simulink model "non_linear_MBS_PF_final"  (R2021b)
settings: Solver=ode15s  StartTime=0  StopTime=SimTime  FixedStep=.00001  RelTol=1e-3
values from neighbouring files (symbols this model uses):
  A_ratio = 1.41    [non_linear_param_MBS_PF_final.m]    (also in non_linear_param_final.m)
  Annulus_area = Bore_area-Rod_area    (= 2356.19449019)    [non_linear_param_MBS_PF_final.m]    (also in non_linear_param_final.m)
  beta_F = 12000e5    (= 1200000000)    [non_linear_param_MBS_PF_final.m]    (also in non_linear_param_final.m)
  Bore_area = pi/4*Bore_diameter^2    (= 3318.30724035)    [non_linear_param_MBS_PF_final.m]    (also in non_linear_param_final.m)
  cyl_c_end = 9e8    (= 900000000)    [non_linear_param_MBS_PF_final.m]    (also in non_linear_param_final.m)
  cyl_c_visc = 1500    [non_linear_param_MBS_PF_final.m]    (also in non_linear_param_final.m)
  cyl_CH_stat = 1/50    (= 0.02)    [non_linear_param_MBS_PF_final.m]    (also in non_linear_param_final.m)
  cyl_F_coul = 75    [non_linear_param_MBS_PF_final.m]    (also in non_linear_param_final.m)
  cyl_FH_stat = 500    [non_linear_param_MBS_PF_final.m]    (also in non_linear_param_final.m)
  cyl_gamma = 250    [non_linear_param_MBS_PF_final.m]    (also in non_linear_param_final.m)
  cyl_k_end = 6e9    (= 6000000000)    [non_linear_param_MBS_PF_final.m]    (also in non_linear_param_final.m)
  cyl_KLi = 0    [non_linear_param_MBS_PF_final.m]    (also in non_linear_param_final.m)
  d_eff_long = sqrt(2*J_sec_long*k_eff_long)    [non_linear_param_MBS_PF_final.m]    (also in non_linear_param_final.m)
  d_eff_short = sqrt(2*J_sec_short*k_eff_short)    [non_linear_param_MBS_PF_final.m]    (also in non_linear_param_final.m)
  DB = 0    [non_linear_param_MBS_PF_final.m]    (also in non_linear_param_final.m = -0.114)
  DR = 1    [non_linear_param_MBS_PF_final.m]    (also in non_linear_param_final.m)
  dr_dcv = 0.7    [non_linear_param_MBS_PF_final.m]    (also in non_linear_param_final.m)
  Eff_Bulk_On = 1    [non_linear_param_MBS_PF_final.m]    (also in non_linear_param_final.m)
  eta_air = 0.7e-2    (= 0.007)    [non_linear_param_MBS_PF_final.m]    (also in non_linear_param_final.m)
  k_cv = 15    [non_linear_param_MBS_PF_final.m]    (also in non_linear_param_final.m)
  k_DCV_PA = 7.85    [non_linear_param_MBS_PF_final.m]    (also in non_linear_param_final.m)
  k_DCV_PB = 7.55    [non_linear_param_MBS_PF_final.m]    (also in non_linear_param_final.m)
  k_DCV_T = 7.85    [non_linear_param_MBS_PF_final.m]    (also in non_linear_param_final.m)
  k_eff_long = E*I/I_sec_long    (= 3050381.94041)    [non_linear_param_MBS_PF_final.m]    (also in non_linear_param_final.m)
  k_eff_short = E*I/I_sec_short    (= 13451432.5427)    [non_linear_param_MBS_PF_final.m]    (also in non_linear_param_final.m)
  k_ocv = 15    [non_linear_param_MBS_PF_final.m]    (also in non_linear_param_final.m)
  K_oil = 1.5e4*0+12000    (= 12000)    [non_linear_param_MBS_PF_final.m]    (also in non_linear_param_final.m)
  k_tanh = 0.005    [non_linear_param_MBS_PF_final.m]    (also in non_linear_param_final.m)
  kappa_air = 1.4    [non_linear_param_MBS_PF_final.m]    (also in non_linear_param_final.m)
  kHP = C*kF_pf_Up    (= 2.90878e-12)    [non_linear_param_MBS_PF_final.m]
  kI = (0.54*ku)/Ti    (= 0.048)    [non_linear_param_MBS_PF_final.m]
  kP = 0.45*ku    (= 0.018)    [non_linear_param_MBS_PF_final.m]
  kq_xS_PB = 4.8946e-04    (= 0.00048946)    [non_linear_param_MBS_PF_final.m]
  KR = 1    [non_linear_param_MBS_PF_final.m]    (also in non_linear_param_final.m)
  mu_ocv = 10    [non_linear_param_MBS_PF_final.m]    (also in non_linear_param_final.m = 3)
  muC = (Bore_diameter^2)/(Bore_diameter^2-Rod_diameter^2)    (= 1.40833333333)    [non_linear_param_MBS_PF_final.m]
  OL_pa = -0.114    [non_linear_param_MBS_PF_final.m]    (also in non_linear_param_final.m)
  OL_pb = 0.114    [non_linear_param_MBS_PF_final.m]    (also in non_linear_param_final.m)
  p0_A = 62.79    [non_linear_param_MBS_PF_final.m]    (also in non_linear_param_final.m)
  p0_A1 = 1.08    [non_linear_param_MBS_PF_final.m]    (also in non_linear_param_final.m)
  p0_B = 1.213    [non_linear_param_MBS_PF_final.m]    (also in non_linear_param_final.m)
  p_cv_fo = 1    [non_linear_param_MBS_PF_final.m]    (also in non_linear_param_final.m)
  p_cv_set = 2.8    [non_linear_param_MBS_PF_final.m]    (also in non_linear_param_final.m)
  p_ocv_fo = 350    [non_linear_param_MBS_PF_final.m]    (also in non_linear_param_final.m)
  p_ocv_set = 196    [non_linear_param_MBS_PF_final.m]    (also in non_linear_param_final.m = 150)
  p_pc_set = 7    [non_linear_param_MBS_PF_final.m]    (also in non_linear_param_final.m)
  p_R = 1.1    [non_linear_param_MBS_PF_final.m]    (also in non_linear_param_final.m)
  p_S = 180    [non_linear_param_MBS_PF_final.m]    (also in non_linear_param_final.m)
  patm = 1.013e5    (= 101300)    [non_linear_param_MBS_PF_final.m]    (also in non_linear_param_final.m)
  Stroke = 500    [non_linear_param_MBS_PF_final.m]    (also in non_linear_param_final.m)
  t0 = 3    [non_linear_param_MBS_PF_final.m]    (also in non_linear_param_final.m = 1)
  tau_ocv = 0.0889*0+0.001    (= 0.001)    [non_linear_param_MBS_PF_final.m]    (also in non_linear_param_final.m)
  tau_pc = tau_ocv    (= 0.001)    [non_linear_param_MBS_PF_final.m]    (also in non_linear_param_final.m)
  tHP = tF_pf_Up/(wn_pf)    (= 0.114613180516)    [non_linear_param_MBS_PF_final.m]
  tStepDown = 4.7*0+7.5    (= 7.5)    [non_linear_param_MBS_PF_final.m]    (also in non_linear_param_final.m = 4.6)
  tStepUp = 10.0    (= 10)    [non_linear_param_MBS_PF_final.m]    (also in non_linear_param_final.m = 6.2)
  tWait = 5.0    (= 5)    [non_linear_param_MBS_PF_final.m]    (also in non_linear_param_final.m = 8)
  UL_at = -0.05    [non_linear_param_MBS_PF_final.m]    (also in non_linear_param_final.m)
  UL_bt = 0.05    [non_linear_param_MBS_PF_final.m]    (also in non_linear_param_final.m)
  Up = 1    [non_linear_param_MBS_PF_final.m]    (also in non_linear_param_final.m)
  uS_sp = -0.4    [non_linear_param_MBS_PF_final.m]    (also in non_linear_param_final.m = -0.55)
  V_CH_A = 1.0e-03    (= 0.001)    [non_linear_param_MBS_PF_final.m]    (also in non_linear_param_final.m)
  V_CH_A1 = 0.1e-03    (= 0.0001)    [non_linear_param_MBS_PF_final.m]    (also in non_linear_param_final.m)
  V_CH_B = 1.25e-03    (= 0.00125)    [non_linear_param_MBS_PF_final.m]    (also in non_linear_param_final.m)
  wn_dcv = 30    [non_linear_param_MBS_PF_final.m]    (also in non_linear_param_final.m)
undefined symbols (not defined in the file or in its folder): sigbuilder_block, smiData, uS0, X, Y, Z
libraries: sm_lib x189, nesl_utility x4, eeGeneralControl x2
(part 3 of 6)

== system non_linear_MBS_PF_final/Comparison Exp1/Large valve opening = 0.5 Down Only ==
  Demux : Demux  Tag=STV Demux  Outputs=9
      settings: DisplayOption=none
  FromWs : FromWorkspace  Tag=STV FromWs  VariableName=tuvar
  Imported_Signal 1 : Outport  Tag=STV Outport  VectorParamsAs1DForOutWhenUnconnected=off
  Imported_Signal 2 : Outport  Tag=STV Outport  Port=2  VectorParamsAs1DForOutWhenUnconnected=off
  Imported_Signal 3 : Outport  Tag=STV Outport  Port=3  VectorParamsAs1DForOutWhenUnconnected=off
  Imported_Signal 4 : Outport  Tag=STV Outport  Port=4  VectorParamsAs1DForOutWhenUnconnected=off
  Imported_Signal 5 : Outport  Tag=STV Outport  Port=5  VectorParamsAs1DForOutWhenUnconnected=off
  Imported_Signal 6 : Outport  Tag=STV Outport  Port=6  VectorParamsAs1DForOutWhenUnconnected=off
  Imported_Signal 7 : Outport  Tag=STV Outport  Port=7  VectorParamsAs1DForOutWhenUnconnected=off
  Imported_Signal 8 : Outport  Tag=STV Outport  Port=8  VectorParamsAs1DForOutWhenUnconnected=off
  Imported_Signal 9 : Outport  Tag=STV Outport  Port=9  VectorParamsAs1DForOutWhenUnconnected=off
  wires:
  Demux/1 -> Imported_Signal 1/1
  FromWs/1 -> Demux/1
  Demux/2 -> Imported_Signal 2/1
  Demux/3 -> Imported_Signal 3/1
  Demux/4 -> Imported_Signal 4/1
  Demux/5 -> Imported_Signal 5/1
  Demux/6 -> Imported_Signal 6/1
  Demux/7 -> Imported_Signal 7/1
  Demux/8 -> Imported_Signal 8/1
  Demux/9 -> Imported_Signal 9/1

== system non_linear_MBS_PF_final/Comparison Exp1/Orginal DOwn ==
  Demux : Demux  Tag=STV Demux  Outputs=9
      settings: DisplayOption=none
  FromWs : FromWorkspace  Tag=STV FromWs  VariableName=tuvar
  Imported_Signal 1 : Outport  Tag=STV Outport  VectorParamsAs1DForOutWhenUnconnected=off
  Imported_Signal 2 : Outport  Tag=STV Outport  Port=2  VectorParamsAs1DForOutWhenUnconnected=off
  Imported_Signal 3 : Outport  Tag=STV Outport  Port=3  VectorParamsAs1DForOutWhenUnconnected=off
  Imported_Signal 4 : Outport  Tag=STV Outport  Port=4  VectorParamsAs1DForOutWhenUnconnected=off
  Imported_Signal 5 : Outport  Tag=STV Outport  Port=5  VectorParamsAs1DForOutWhenUnconnected=off
  Imported_Signal 6 : Outport  Tag=STV Outport  Port=6  VectorParamsAs1DForOutWhenUnconnected=off
  Imported_Signal 7 : Outport  Tag=STV Outport  Port=7  VectorParamsAs1DForOutWhenUnconnected=off
  Imported_Signal 8 : Outport  Tag=STV Outport  Port=8  VectorParamsAs1DForOutWhenUnconnected=off
  Imported_Signal 9 : Outport  Tag=STV Outport  Port=9  VectorParamsAs1DForOutWhenUnconnected=off
  wires:
  Demux/1 -> Imported_Signal 1/1
  FromWs/1 -> Demux/1
  Demux/2 -> Imported_Signal 2/1
  Demux/3 -> Imported_Signal 3/1
  Demux/4 -> Imported_Signal 4/1
  Demux/5 -> Imported_Signal 5/1
  Demux/6 -> Imported_Signal 6/1
  Demux/7 -> Imported_Signal 7/1
  Demux/8 -> Imported_Signal 8/1
  Demux/9 -> Imported_Signal 9/1

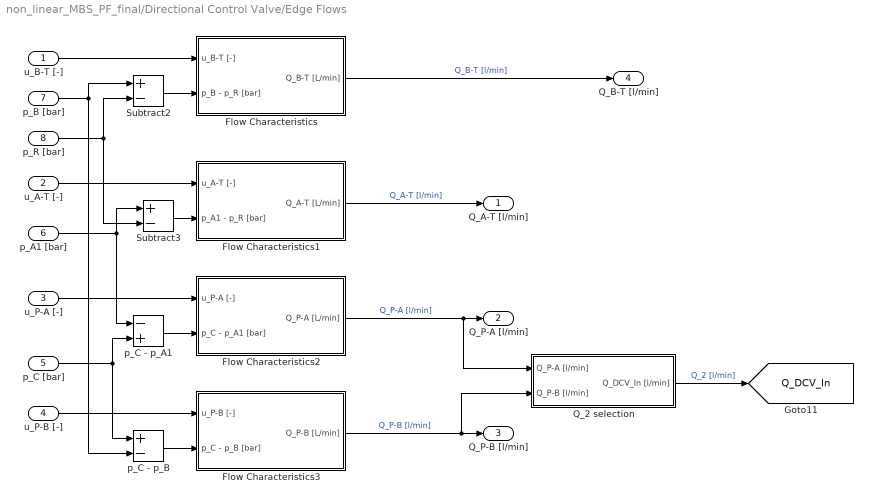
== system non_linear_MBS_PF_final/Directional Control Valve/Edge Flows ==
  u_B-T [-] : Inport
  u_A-T [-] : Inport  Port=2
  u_P-A [-] : Inport  Port=3
  u_P-B [-] : Inport  Port=4
  p_C [bar] : Inport  Port=5
  p_A1 [bar] : Inport  Port=6
  p_B [bar] : Inport  Port=7
  p_R [bar] : Inport  Port=8
  Flow Characteristics : Subsystem
  Flow Characteristics1 : Subsystem
  Flow Characteristics2 : Subsystem
  Flow Characteristics3 : Subsystem
  Q_2 selection : Subsystem
  Subtract2 : Sum  Inputs=+-
  Subtract3 : Sum  Inputs=+-
  p_C - p_A1 : Sum  Inputs=-+
  p_C - p_B : Sum  Inputs=+-
  Q_A-T [l/min] : Outport  VectorParamsAs1DForOutWhenUnconnected=off
  Q_P-A [l/min] : Outport  Port=2  VectorParamsAs1DForOutWhenUnconnected=off
  Q_P-B [l/min] : Outport  Port=3  VectorParamsAs1DForOutWhenUnconnected=off
  Q_B-T [l/min] : Outport  Port=4  VectorParamsAs1DForOutWhenUnconnected=off
  tag scope: Q_DCV_In=global
  wires:
  p_C [bar]/1 -> p_C - p_A1/2, p_C - p_B/1
  u_A-T [-]/1 -> Flow Characteristics1/1(u_A-T [-])
  p_A1 [bar]/1 -> Subtract3/1, p_C - p_A1/1
  p_B [bar]/1 -> Subtract2/1, p_C - p_B/2
  p_C - p_B/1 -> Flow Characteristics3/2(p_C - p_B [bar])
  u_P-A [-]/1 -> Flow Characteristics2/1(u_P-A [-])
  p_C - p_A1/1 -> Flow Characteristics2/2(p_C - p_A1 [bar])
  u_P-B [-]/1 -> Flow Characteristics3/1(u_P-B [-])
  p_R [bar]/1 -> Subtract3/2, Subtract2/2
  Flow Characteristics1/1(Q_A-T [L/min]) -> Q_A-T [l/min]/1  "Q_A-T [l/min]"
  Flow Characteristics/1(Q_B-T [L/min]) -> Q_B-T [l/min]/1  "Q_B-T [l/min]"
  Q_2 selection/1(Q_DCV_In [l/min]) -> [Q_DCV_In]  "Q_2 [l/min]"
  Flow Characteristics3/1(Q_P-B [L/min]) -> Q_2 selection/2(Q_P-B [l/min]), Q_P-B [l/min]/1  "Q_P-B [l/min]"
  Flow Characteristics2/1(Q_P-A [L/min]) -> Q_2 selection/1(Q_P-A [l/min]), Q_P-A [l/min]/1  "Q_P-A [l/min]"
  Subtract2/1 -> Flow Characteristics/2(p_B - p_R [bar])
  u_B-T [-]/1 -> Flow Characteristics/1(u_B-T [-])
  Subtract3/1 -> Flow Characteristics1/2(p_A1 - p_R [bar])

== system non_linear_MBS_PF_final/Directional Control Valve/Edge Openings ==
  u_dcv [-] : Inport
  Edge A-T : Subsystem
  Edge B-T : Subsystem
  Edge P-A : Subsystem
  Edge P-B : Subsystem
  u_B-T [-] : Outport  VectorParamsAs1DForOutWhenUnconnected=off
  u_A-T [-] : Outport  Port=2  VectorParamsAs1DForOutWhenUnconnected=off
  u_P-A [-] : Outport  Port=3  VectorParamsAs1DForOutWhenUnconnected=off
  u_P-B [-] : Outport  Port=4  VectorParamsAs1DForOutWhenUnconnected=off
  wires:
  Edge A-T/1(u_A-T [-]) -> u_A-T [-]/1  "u_A-T [-]"
  Edge B-T/1(u_B-T [-]) -> u_B-T [-]/1  "u_B-T [-]"
  u_dcv [-]/1 -> Edge P-A/1(u_dcv [-]), Edge P-B/1(u_dcv [-]), Edge A-T/1(u_dcv [-]), Edge B-T/1(u_dcv [-])  "[-]"
  Edge P-A/1(u_P-A [-]) -> u_P-A [-]/1  "u_P-A [-]"
  Edge P-B/1(u_P-B [-]) -> u_P-B [-]/1  "u_P-B [-]"

== system non_linear_MBS_PF_final/Directional Control Valve/Load-Sensing Pressure ==
  pB : Inport
  pA1 : Inport  Port=2
  uDCV : Inport  Port=3
  Constant : Constant  Value=0
  Switch : Switch  Criteria=u2 ~= 0
  Switch1 : Switch  Criteria=u2 > Threshold
  pLS : Outport  VectorParamsAs1DForOutWhenUnconnected=off
  wires:
  pA1/1 -> Switch1/3
  Constant/1 -> Switch/3
  Switch1/1 -> Switch/1
  Switch/1 -> pLS/1
  uDCV/1 -> Switch1/2, Switch/2
  pB/1 -> Switch1/1

== system non_linear_MBS_PF_final/Directional Control Valve/Valve Dynamics ==
  note: 2nd order TF for
      | DCV dynamics
  u_dcv_ref [-] : Inport
  2nd order TF : TransferFcn  Denominator="[1/(wn_dcv^2) 2*dr_dcv/wn_dcv  1]"
  u_dcv [-] : Outport  VectorParamsAs1DForOutWhenUnconnected=off
  tag scope: u_dcv=global
  wires:
  u_dcv_ref [-]/1 -> 2nd order TF/1  "u_dcv_ref [-]"
  2nd order TF/1 -> [u_dcv], u_dcv [-]/1  "u_dcv [-]"

== system non_linear_MBS_PF_final/Monitoring/Subsystem ==
  Constant1 : Constant  Value=A_ratio
  Divide3 : Product  Inputs=**
  Gain15 : Gain  Gain=-1
  Q_A exp [L/min] : Outport  VectorParamsAs1DForOutWhenUnconnected=off
  wires:
  [Q_B_exp] -> Divide3/1
  Gain15/1 -> Q_A exp [L/min]/1  "Q_A exp [L/min]"
  Constant1/1 -> Divide3/2
  Divide3/1 -> Gain15/1

== system non_linear_MBS_PF_final/Over-Centre Valve/Capasitance ==
  Bulk Modulus : Subsystem
  Constant : Constant  Value=V_CH_A1
  Divide : Product  Inputs=*/
  "CH_A1 " : Outport  VectorParamsAs1DForOutWhenUnconnected=off
  wires:
  Bulk Modulus/1(K_oil) -> Divide/2  "[bar]"
  Constant/1 -> Divide/1  "[m^3]"
  Divide/1 -> "CH_A1 "/1

== system non_linear_MBS_PF_final/Over-Centre Valve/Check Valve ==
  p_A [bar] : Inport
  p_A1 [bar] : Inport  Port=2
  Abs : Abs  ZeroCross=off
  Constant : Constant  Value=k_cv
  Product : Product  Inputs=****
  Saturation : Saturate  UpperLimit=1  LowerLimit=0  ZeroCross=off
  Sign : Subsystem
  Sqrt : Sqrt  SaturateOnIntegerOverflow=on
  Sum : Sum  Inputs=+-
  Sum1 : Sum  Inputs=+-
  division by p_cv_fo : Gain  Gain=1/p_cv_fo
  p_cv_set [bar] : Constant  Value=p_cv_set
  Q_cv [l/min] : Outport  VectorParamsAs1DForOutWhenUnconnected=off
  wires:
  p_cv_set [bar]/1 -> Sum1/2
  Sum/1 -> Abs/1, Sum1/1, Sign/1(In)  "p2-p3 [bar]"
  p_A [bar]/1 -> Sum/2
  p_A1 [bar]/1 -> Sum/1
  division by p_cv_fo/1 -> Saturation/1  "u_cv [-]"
  Sum1/1 -> division by p_cv_fo/1
  Constant/1 -> Product/1  "[l/min/bar^0.5]"
  Sqrt/1 -> Product/2  "[bar^0.5]"
  Product/1 -> Q_cv [l/min]/1
  Abs/1 -> Sqrt/1
  Sign/1(Out) -> Product/4
  Saturation/1 -> Product/3

== system non_linear_MBS_PF_final/Over-Centre Valve/Over-Centre Valve ==
  u_ocv [-] : Inport
  p_A [bar] : Inport  Port=2
  p_A1 [bar] : Inport  Port=3
  Abs : Abs  ZeroCross=off
  Product : Product  Inputs=****
  Sign : Subsystem
  Sqrt : Sqrt  SaturateOnIntegerOverflow=on
  k_ocv [l/min/bar^0.5] : Constant  Value=k_ocv
  p3-p2 : Sum  Inputs=+-
  "[l/min]" : Outport  VectorParamsAs1DForOutWhenUnconnected=off
  wires:
  k_ocv [l/min/bar^0.5]/1 -> Product/3
  p3-p2/1 -> Sign/1(In), Abs/1  "p3-p2 [bar]"
  Sqrt/1 -> Product/2  "[bar^0.5]"
  Product/1 -> "[l/min]"/1  "[l/min]"
  Sign/1(Out) -> Product/4
  Abs/1 -> Sqrt/1
  u_ocv [-]/1 -> Product/1
  p_A1 [bar]/1 -> p3-p2/2
  p_A [bar]/1 -> p3-p2/1

== system non_linear_MBS_PF_final/Over-Centre Valve/Pressure Equillibrium ==
  p_B [bar] : Inport
  p_A [bar] : Inport  Port=2
  Division by p_ocv_fo [bar] : Gain  Gain=1/p_ocv_fo
  Pilot ratio [-] : Gain  Gain=mu_ocv
  Saturation : Saturate  UpperLimit=1  LowerLimit=0  ZeroCross=off
  Scope : Scope
  Sum : Sum  Inputs=++-
  Valve Dynamics : TransferFcn  Denominator=[tau_ocv 1]
  p_ocv_set [bar] : Constant  Value=p_ocv_set
  u_ocv [-] : Outport  VectorParamsAs1DForOutWhenUnconnected=off
  wires:
  Sum/1 -> Division by p_ocv_fo [bar]/1  "[bar]"
  Pilot ratio [-]/1 -> Sum/1
  p_ocv_set [bar]/1 -> Sum/3
  p_B [bar]/1 -> Pilot ratio [-]/1
  p_A [bar]/1 -> Sum/2
  Division by p_ocv_fo [bar]/1 -> Saturation/1  "u_ocv_ref [-]"
  Saturation/1 -> Valve Dynamics/1
  Valve Dynamics/1 -> Scope/1, u_ocv [-]/1  "[-]"

== system non_linear_MBS_PF_final/Subsystem/CH Bore Side ==
  x [m] : Inport
  Bulk Modulus : Subsystem
  Constant4 : Constant  Value=V_CH_A
  Divide2 : Product  Inputs=*/
  Gain1 : Gain  Gain=Bore_area
  Gain2 : Gain  Gain=1/(10)^9
  Gain3 : Gain  Gain=1000
  Sum3 : Sum
  CH_A : Outport  VectorParamsAs1DForOutWhenUnconnected=off
  wires:
  Sum3/1 -> Divide2/1  "[m^3]"
  x [m]/1 -> Gain3/1
  Constant4/1 -> Sum3/1  "[m^3]"
  Gain1/1 -> Gain2/1  "[mm^3]"
  Gain3/1 -> Gain1/1  "[mm]"
  Gain2/1 -> Sum3/2  "[m^3]"
  Divide2/1 -> CH_A/1
  Bulk Modulus/1(K_oil) -> Divide2/2  "[bar]"

== system non_linear_MBS_PF_final/Subsystem/CH Rod Side ==
  x [m] : Inport
  Bulk Modulus : Subsystem
  Constant1 : Constant  Value=Stroke
  Constant4 : Constant  Value=V_CH_B
  Divide2 : Product  Inputs=*/
  Gain1 : Gain  Gain=Annulus_area
  Gain2 : Gain  Gain=1/(10)^9
  Gain4 : Gain  Gain=1000
  Sum2 : Sum  Inputs=+-
  Sum3 : Sum
  CH_B : Outport  VectorParamsAs1DForOutWhenUnconnected=off
  wires:
  Sum3/1 -> Divide2/1  "[m^3]"
  Constant1/1 -> Sum2/1  "[mm]"
  x [m]/1 -> Gain4/1
  Sum2/1 -> Gain1/1
  Constant4/1 -> Sum3/1  "[m^3]"
  Gain1/1 -> Gain2/1  "[mm^3]"
  Gain4/1 -> Sum2/2  "[mm]"
  Gain2/1 -> Sum3/2  "[m^3]"
  Divide2/1 -> CH_B/1
  Bulk Modulus/1(K_oil) -> Divide2/2

== system non_linear_MBS_PF_final/Subsystem/Cylinder forces ==
  v_cyl [m/s] : Inport
  F_hyd [N] : Inport  Port=2
  x _cyl [m] : Inport  Port=3
  Add : Sum  Inputs=+-+-
  Constant : Constant  Value=0
  Lower Stroke Limit : Subsystem
  Manual Switch : ManualSwitch
  Stribeck Friction1 : Subsystem
  Upper Stroke Limit : Subsystem
  F_piston [N] : Outport  VectorParamsAs1DForOutWhenUnconnected=off
  wires:
  Lower Stroke Limit/1(F_cyl_contact [N]) -> Add/3
  Upper Stroke Limit/1(F_cyl_contact [N]) -> Add/2
  x _cyl [m]/1 -> Upper Stroke Limit/1(x_cyl [m]), Lower Stroke Limit/1(x_cyl [m])
  Add/1 -> F_piston [N]/1  "Bm_Cyl_F"
  Stribeck Friction1/1(F [N]) -> Manual Switch/1
  v_cyl [m/s]/1 -> Stribeck Friction1/1(v_cyl [m/s]), Lower Stroke Limit/2(v_cyl [m]), Upper Stroke Limit/2(v_cyl [m/s])
  Manual Switch/1 -> Add/4
  F_hyd [N]/1 -> Add/1
  Constant/1 -> Manual Switch/2

== system non_linear_MBS_PF_final/Subsystem/Internal Leakages ==
  Dp [bar] : Inport  PortDimensions=1
  Conv.  1 : Gain  Gain=cyl_KLi
  Product : Product  RndMeth=Zero
  Sign : Signum
  Q_L [L/min] : Outport  PortDimensions=1  VectorParamsAs1DForOutWhenUnconnected=off
  wires:
  Dp [bar]/1 -> Conv.  1/1, Sign/1
  Product/1 -> Q_L [L/min]/1
  Conv.  1/1 -> Product/1
  Sign/1 -> Product/2  "[-]"

== system non_linear_MBS_PF_final/Subsystem1/VCC_Step_Function_Validation ==
  Enable : Inport  OutDataTypeStr=boolean
  u_SP : Inport  Port=2
  uS0 : Inport  Port=3
  DB : Inport  Port=4
  CyclicTime : Inport  Port=5
  t0 : Inport  Port=6
  tHold1 : Inport  Port=7
  tStep : Inport  Port=8
  tHold2 : Inport  Port=9
  Constant : Constant  Value=0
  StepFunction : MATLAB Function  TreatAsAtomicUnit=on
  Switch : Switch  Criteria=u2 > Threshold
  u_ref : Outport  VectorParamsAs1DForOutWhenUnconnected=off
  EndTime : Outport  Port=2  VectorParamsAs1DForOutWhenUnconnected=off
  wires:
  u_SP/1 -> StepFunction/1(u_SP)
  tStep/1 -> StepFunction/7(t2)
  tHold1/1 -> StepFunction/6(t1)
  t0/1 -> StepFunction/5(t0)
  CyclicTime/1 -> StepFunction/4(t)
  StepFunction/2(T) -> EndTime/1
  uS0/1 -> StepFunction/2(uS0)
  tHold2/1 -> StepFunction/8(t3)
  DB/1 -> StepFunction/3(DB)
  StepFunction/1(y) -> Switch/1
  Switch/1 -> u_ref/1
  Constant/1 -> Switch/3
  Enable/1 -> Switch/2

== system non_linear_MBS_PF_final/Subsystem2/Efficiency v1 ==
  Pin : Inport
  Pout : Inport  Port=2
  1 : Scope
  2 : Scope
  3 : Scope
  Fcn4 : Fcn  Expr=u(2)/(u(1)+eps)
  Integrator2 : Integrator  LowerSaturationLimit=0
  Mux9 : Mux  Inputs=2
  Saturation3 : Saturate  UpperLimit=inf  LowerLimit=0
  Saturation4 : Saturate  UpperLimit=1  LowerLimit=0
  eta_io : Outport  VectorParamsAs1DForOutWhenUnconnected=off
  wires:
  Pin/1 -> Mux9/1
  Pout/1 -> Mux9/2
  Mux9/1 -> Saturation3/1, 1/1
  Saturation4/1 -> eta_io/1
  Fcn4/1 -> Saturation4/1
  Integrator2/1 -> Fcn4/1, 3/1
  Saturation3/1 -> Integrator2/1, 2/1

== system non_linear_MBS_PF_final/Test/Arm_ballast_big_4_RIGID ==
  ReferenceFrame : sm_lib/Frames and Transforms/Reference Frame
  Rigid Transform2 : sm_lib/Frames and Transforms/Rigid Transform  TranslationMethod=Cartesian  TranslationStandardOffset=0  TranslationLengthUnit=mm  TranslationStandardAxis=+Z  TranslationCartesianOffset=[0 6.5 -(609.43814785-105.00)]  TranslationROffset=0  TranslationROffsetUnits=m  TranslationZOffset=0  TranslationZOffsetUnits=m  TranslationThetaOffset=0  TranslationThetaOffsetUnits=deg  RotationMethod=ArbitraryAxis  RotationAngleUnits=deg  RotationStandardAxis=+Z  RotationAngle=0  RotationArbitraryAxis=[0 1 0]  FollAlignAxisA=+X  BaseAlignAxisA=+Y  FollAlignAxisB=+Y  BaseAlignAxisB=+Z  RotationSequenceAxes=FollowerAxes  RotationSequence=XYX  RotationSequenceAngles=[0 0 0]  RotationSequenceAnglesUnits=deg  RotationMatrix=[1 0 0; 0 1 0; 0 0 1]
  Rigid Transform3 : sm_lib/Frames and Transforms/Rigid Transform  TranslationMethod=Cartesian  TranslationStandardOffset=0  TranslationLengthUnit=mm  TranslationStandardAxis=+Z  TranslationCartesianOffset=[0 0 105]  TranslationROffset=0  TranslationROffsetUnits=m  TranslationZOffset=0  TranslationZOffsetUnits=m  TranslationThetaOffset=0  TranslationThetaOffsetUnits=deg  RotationMethod=ArbitraryAxis  RotationAngleUnits=deg  RotationStandardAxis=+Z  RotationAngle=0  RotationArbitraryAxis=[0 1 0]  FollAlignAxisA=+X  BaseAlignAxisA=+Y  FollAlignAxisB=+Y  BaseAlignAxisB=+Z  RotationSequenceAxes=FollowerAxes  RotationSequence=XYX  RotationSequenceAngles=[0 0 0]  RotationSequenceAnglesUnits=deg  RotationMatrix=[1 0 0; 0 1 0; 0 0 1]
  Solid : sm_lib/Body Elements/File Solid  ExtGeomFileName=Arm_ballast_big_Default_sldprt.STEP  UnitType=FromFile  ExtGeomFileUnits=m  StepReaderType=OCC  ExportConvexHullGeometry=off  InertiaType=CalculateFromGeometry  Mass=327.5000  MassUnits=kg  CenterOfMass=smiData.Solid(23).CoM  CenterOfMassUnits=mm  MomentsOfInertia=smiData.Solid(23).MoI  MomentsOfInertiaUnits=kg*mm^2  ProductsOfInertia=smiData.Solid(23).PoI  ProductsOfInertiaUnits=kg*mm^2  BasedOnType=Mass  Density=10000  DensityUnits=kg/m^3  DoExposeReferenceFrame=on  SerializedFrames=<Frames/>  GraphicType=FromGeometry  MarkerShape=Sphere  MarkerSize=10  GraphicVisPropType=SimpleVisualProperties  GraphicDiffuseColor=[1.0 0.8 0.0]  GraphicSpecularColor=[0.5 0.5 0.5 1.0]  GraphicAmbientColor=[0.15 0.15 0.15 1.0]  GraphicEmissiveColor=[0.0 0.0 0.0 1.0]  GraphicShininess=75  GraphicOpacity=smiData.Solid(23).opacity
  F : PMIOPort  Side=Left
  F1 : PMIOPort  Port=2  Side=Right
  connections:
  ReferenceFrame/RConn1 -- Rigid Transform2/LConn1 -- Rigid Transform3/LConn1 -- Solid/RConn1
  F/RConn1 -- Rigid Transform2/RConn1
  Rigid Transform3/RConn1 -- F1/RConn1

== system non_linear_MBS_PF_final/Test/Arm_long_1_RIGID ==
  ReferenceFrame2 : sm_lib/Frames and Transforms/Reference Frame
  Rigid Transform : sm_lib/Frames and Transforms/Rigid Transform  TranslationMethod=Cartesian  TranslationStandardOffset=0  TranslationLengthUnit=m  TranslationStandardAxis=+Z  TranslationCartesianOffset=[0 0 0.6094]  TranslationROffset=0  TranslationROffsetUnits=m  TranslationZOffset=0  TranslationZOffsetUnits=m  TranslationThetaOffset=0  TranslationThetaOffsetUnits=deg  RotationMethod=ArbitraryAxis  RotationAngleUnits=deg  RotationStandardAxis=+Z  RotationAngle=90  RotationArbitraryAxis=[0 1 0]  FollAlignAxisA=+X  BaseAlignAxisA=+Y  FollAlignAxisB=+Y  BaseAlignAxisB=+Z  RotationSequenceAxes=FollowerAxes  RotationSequence=XYX  RotationSequenceAngles=[0 0 0]  RotationSequenceAnglesUnits=deg  RotationMatrix=[1 0 0; 0 1 0; 0 0 1]
  Rigid Transform3 : sm_lib/Frames and Transforms/Rigid Transform  TranslationMethod=Cartesian  TranslationStandardOffset=0  TranslationLengthUnit=m  TranslationStandardAxis=+Z  TranslationCartesianOffset=[0 0 0]  TranslationROffset=0  TranslationROffsetUnits=m  TranslationZOffset=0  TranslationZOffsetUnits=m  TranslationThetaOffset=0  TranslationThetaOffsetUnits=deg  RotationMethod=ArbitraryAxis  RotationAngleUnits=deg  RotationStandardAxis=+Z  RotationAngle=-90  RotationArbitraryAxis=[0 1 0]  FollAlignAxisA=+X  BaseAlignAxisA=+Y  FollAlignAxisB=+Y  BaseAlignAxisB=+Z  RotationSequenceAxes=FollowerAxes  RotationSequence=XYX  RotationSequenceAngles=[0 0 0]  RotationSequenceAnglesUnits=deg  RotationMatrix=[1 0 0; 0 1 0; 0 0 1]
  Solid : sm_lib/Body Elements/File Solid  ExtGeomFileName=Arm_long_Default_sldprt.STEP  UnitType=FromFile  ExtGeomFileUnits=m  StepReaderType=OCC  ExportConvexHullGeometry=off  InertiaType=CalculateFromGeometry  Mass=13.5799  MassUnits=kg  CenterOfMass=smiData.Solid(19).CoM  CenterOfMassUnits=mm  MomentsOfInertia=smiData.Solid(19).MoI  MomentsOfInertiaUnits=kg*mm^2  ProductsOfInertia=smiData.Solid(19).PoI  ProductsOfInertiaUnits=kg*mm^2  BasedOnType=Mass  Density=7800  DensityUnits=kg/m^3  DoExposeReferenceFrame=on  SerializedFrames=<Frames/>  GraphicType=FromGeometry  MarkerShape=Sphere  MarkerSize=10  GraphicVisPropType=SimpleVisualProperties  GraphicDiffuseColor=[0.2 0.6 0.0]  GraphicSpecularColor=[0.5 0.5 0.5 1.0]  GraphicAmbientColor=[0.15 0.15 0.15 1.0]  GraphicEmissiveColor=[0.0 0.0 0.0 1.0]  GraphicShininess=75  GraphicOpacity=smiData.Solid(19).opacity
  B : PMIOPort  Side=Left
  F : PMIOPort  Port=2  Side=Right
  connections:
  Rigid Transform3/LConn1 -- B/RConn1
  ReferenceFrame2/RConn1 -- Solid/RConn1 -- Rigid Transform3/RConn1 -- Rigid Transform/LConn1
  F/RConn1 -- Rigid Transform/RConn1

== system non_linear_MBS_PF_final/Test/Arm_long_2_RIGID ==
  ReferenceFrame2 : sm_lib/Frames and Transforms/Reference Frame
  Rigid Transform : sm_lib/Frames and Transforms/Rigid Transform  TranslationMethod=Cartesian  TranslationStandardOffset=0  TranslationLengthUnit=mm  TranslationStandardAxis=+Z  TranslationCartesianOffset=[0 0 609.43814785]  TranslationROffset=0  TranslationROffsetUnits=m  TranslationZOffset=0  TranslationZOffsetUnits=m  TranslationThetaOffset=0  TranslationThetaOffsetUnits=deg  RotationMethod=ArbitraryAxis  RotationAngleUnits=deg  RotationStandardAxis=+Z  RotationAngle=90  RotationArbitraryAxis=[0 1 0]  FollAlignAxisA=+X  BaseAlignAxisA=+Y  FollAlignAxisB=+Y  BaseAlignAxisB=+Z  RotationSequenceAxes=FollowerAxes  RotationSequence=XYX  RotationSequenceAngles=[0 0 0]  RotationSequenceAnglesUnits=deg  RotationMatrix=[1 0 0; 0 1 0; 0 0 1]
  Rigid Transform3 : sm_lib/Frames and Transforms/Rigid Transform  TranslationMethod=Cartesian  TranslationStandardOffset=0  TranslationLengthUnit=m  TranslationStandardAxis=+Z  TranslationCartesianOffset=[0 0 0]  TranslationROffset=0  TranslationROffsetUnits=m  TranslationZOffset=0  TranslationZOffsetUnits=m  TranslationThetaOffset=0  TranslationThetaOffsetUnits=deg  RotationMethod=ArbitraryAxis  RotationAngleUnits=deg  RotationStandardAxis=+Z  RotationAngle=-90  RotationArbitraryAxis=[0 1 0]  FollAlignAxisA=+X  BaseAlignAxisA=+Y  FollAlignAxisB=+Y  BaseAlignAxisB=+Z  RotationSequenceAxes=FollowerAxes  RotationSequence=XYX  RotationSequenceAngles=[0 0 0]  RotationSequenceAnglesUnits=deg  RotationMatrix=[1 0 0; 0 1 0; 0 0 1]
  Solid : sm_lib/Body Elements/File Solid  ExtGeomFileName=Arm_long_Default_sldprt.STEP  UnitType=FromFile  ExtGeomFileUnits=m  StepReaderType=OCC  ExportConvexHullGeometry=off  InertiaType=CalculateFromGeometry  Mass=13.5799  MassUnits=kg  CenterOfMass=smiData.Solid(19).CoM  CenterOfMassUnits=mm  MomentsOfInertia=smiData.Solid(19).MoI  MomentsOfInertiaUnits=kg*mm^2  ProductsOfInertia=smiData.Solid(19).PoI  ProductsOfInertiaUnits=kg*mm^2  BasedOnType=Mass  Density=7800  DensityUnits=kg/m^3  DoExposeReferenceFrame=on  SerializedFrames=<Frames/>  GraphicType=FromGeometry  MarkerShape=Sphere  MarkerSize=10  GraphicVisPropType=SimpleVisualProperties  GraphicDiffuseColor=[0.2 0.6 0.0]  GraphicSpecularColor=[0.5 0.5 0.5 1.0]  GraphicAmbientColor=[0.15 0.15 0.15 1.0]  GraphicEmissiveColor=[0.0 0.0 0.0 1.0]  GraphicShininess=75  GraphicOpacity=smiData.Solid(19).opacity
  B : PMIOPort  Side=Left
  F : PMIOPort  Port=2  Side=Right
  connections:
  Rigid Transform3/LConn1 -- B/RConn1
  ReferenceFrame2/RConn1 -- Solid/RConn1 -- Rigid Transform3/RConn1 -- Rigid Transform/LConn1
  F/RConn1 -- Rigid Transform/RConn1

== system non_linear_MBS_PF_final/Test/Arm_long_3_RIGID ==
  ReferenceFrame2 : sm_lib/Frames and Transforms/Reference Frame
  Rigid Transform : sm_lib/Frames and Transforms/Rigid Transform  TranslationMethod=Cartesian  TranslationStandardOffset=0  TranslationLengthUnit=mm  TranslationStandardAxis=+Z  TranslationCartesianOffset=[0 0 609.43814785]  TranslationROffset=0  TranslationROffsetUnits=m  TranslationZOffset=0  TranslationZOffsetUnits=m  TranslationThetaOffset=0  TranslationThetaOffsetUnits=deg  RotationMethod=ArbitraryAxis  RotationAngleUnits=deg  RotationStandardAxis=+Z  RotationAngle=90  RotationArbitraryAxis=[0 1 0]  FollAlignAxisA=+X  BaseAlignAxisA=+Y  FollAlignAxisB=+Y  BaseAlignAxisB=+Z  RotationSequenceAxes=FollowerAxes  RotationSequence=XYX  RotationSequenceAngles=[0 0 0]  RotationSequenceAnglesUnits=deg  RotationMatrix=[1 0 0; 0 1 0; 0 0 1]
  Rigid Transform3 : sm_lib/Frames and Transforms/Rigid Transform  TranslationMethod=Cartesian  TranslationStandardOffset=0  TranslationLengthUnit=m  TranslationStandardAxis=+Z  TranslationCartesianOffset=[0 0 0]  TranslationROffset=0  TranslationROffsetUnits=m  TranslationZOffset=0  TranslationZOffsetUnits=m  TranslationThetaOffset=0  TranslationThetaOffsetUnits=deg  RotationMethod=ArbitraryAxis  RotationAngleUnits=deg  RotationStandardAxis=+Z  RotationAngle=-90  RotationArbitraryAxis=[0 1 0]  FollAlignAxisA=+X  BaseAlignAxisA=+Y  FollAlignAxisB=+Y  BaseAlignAxisB=+Z  RotationSequenceAxes=FollowerAxes  RotationSequence=XYX  RotationSequenceAngles=[0 0 0]  RotationSequenceAnglesUnits=deg  RotationMatrix=[1 0 0; 0 1 0; 0 0 1]
  Solid : sm_lib/Body Elements/File Solid  ExtGeomFileName=Arm_long_Default_sldprt.STEP  UnitType=FromFile  ExtGeomFileUnits=m  StepReaderType=OCC  ExportConvexHullGeometry=off  InertiaType=CalculateFromGeometry  Mass=13.5799  MassUnits=kg  CenterOfMass=smiData.Solid(19).CoM  CenterOfMassUnits=mm  MomentsOfInertia=smiData.Solid(19).MoI  MomentsOfInertiaUnits=kg*mm^2  ProductsOfInertia=smiData.Solid(19).PoI  ProductsOfInertiaUnits=kg*mm^2  BasedOnType=Mass  Density=7800  DensityUnits=kg/m^3  DoExposeReferenceFrame=on  SerializedFrames=<Frames/>  GraphicType=FromGeometry  MarkerShape=Sphere  MarkerSize=10  GraphicVisPropType=SimpleVisualProperties  GraphicDiffuseColor=[0.2 0.6 0.0]  GraphicSpecularColor=[0.5 0.5 0.5 1.0]  GraphicAmbientColor=[0.15 0.15 0.15 1.0]  GraphicEmissiveColor=[0.0 0.0 0.0 1.0]  GraphicShininess=75  GraphicOpacity=smiData.Solid(19).opacity
  B : PMIOPort  Side=Left
  F : PMIOPort  Port=2  Side=Right
  connections:
  Rigid Transform3/LConn1 -- B/RConn1
  ReferenceFrame2/RConn1 -- Solid/RConn1 -- Rigid Transform3/RConn1 -- Rigid Transform/LConn1
  F/RConn1 -- Rigid Transform/RConn1

== system non_linear_MBS_PF_final/Test/Arm_long_4_RIGID ==
  ReferenceFrame2 : sm_lib/Frames and Transforms/Reference Frame
  Rigid Transform : sm_lib/Frames and Transforms/Rigid Transform  TranslationMethod=Cartesian  TranslationStandardOffset=0  TranslationLengthUnit=mm  TranslationStandardAxis=+Z  TranslationCartesianOffset=[0 0 609.43814785]  TranslationROffset=0  TranslationROffsetUnits=m  TranslationZOffset=0  TranslationZOffsetUnits=m  TranslationThetaOffset=0  TranslationThetaOffsetUnits=deg  RotationMethod=ArbitraryAxis  RotationAngleUnits=deg  RotationStandardAxis=+Z  RotationAngle=90  RotationArbitraryAxis=[0 1 0]  FollAlignAxisA=+X  BaseAlignAxisA=+Y  FollAlignAxisB=+Y  BaseAlignAxisB=+Z  RotationSequenceAxes=FollowerAxes  RotationSequence=XYX  RotationSequenceAngles=[0 0 0]  RotationSequenceAnglesUnits=deg  RotationMatrix=[1 0 0; 0 1 0; 0 0 1]
  Rigid Transform3 : sm_lib/Frames and Transforms/Rigid Transform  TranslationMethod=Cartesian  TranslationStandardOffset=0  TranslationLengthUnit=m  TranslationStandardAxis=+Z  TranslationCartesianOffset=[0 0 0]  TranslationROffset=0  TranslationROffsetUnits=m  TranslationZOffset=0  TranslationZOffsetUnits=m  TranslationThetaOffset=0  TranslationThetaOffsetUnits=deg  RotationMethod=ArbitraryAxis  RotationAngleUnits=deg  RotationStandardAxis=+Z  RotationAngle=-90  RotationArbitraryAxis=[0 1 0]  FollAlignAxisA=+X  BaseAlignAxisA=+Y  FollAlignAxisB=+Y  BaseAlignAxisB=+Z  RotationSequenceAxes=FollowerAxes  RotationSequence=XYX  RotationSequenceAngles=[0 0 0]  RotationSequenceAnglesUnits=deg  RotationMatrix=[1 0 0; 0 1 0; 0 0 1]
  Solid : sm_lib/Body Elements/File Solid  ExtGeomFileName=Arm_long_Default_sldprt.STEP  UnitType=FromFile  ExtGeomFileUnits=m  StepReaderType=OCC  ExportConvexHullGeometry=off  InertiaType=CalculateFromGeometry  Mass=13.5799  MassUnits=kg  CenterOfMass=smiData.Solid(19).CoM  CenterOfMassUnits=mm  MomentsOfInertia=smiData.Solid(19).MoI  MomentsOfInertiaUnits=kg*mm^2  ProductsOfInertia=smiData.Solid(19).PoI  ProductsOfInertiaUnits=kg*mm^2  BasedOnType=Mass  Density=7800  DensityUnits=kg/m^3  DoExposeReferenceFrame=on  SerializedFrames=<Frames/>  GraphicType=FromGeometry  MarkerShape=Sphere  MarkerSize=10  GraphicVisPropType=SimpleVisualProperties  GraphicDiffuseColor=[0.2 0.6 0.0]  GraphicSpecularColor=[0.5 0.5 0.5 1.0]  GraphicAmbientColor=[0.15 0.15 0.15 1.0]  GraphicEmissiveColor=[0.0 0.0 0.0 1.0]  GraphicShininess=75  GraphicOpacity=smiData.Solid(19).opacity
  B : PMIOPort  Side=Left
  F : PMIOPort  Port=2  Side=Right
  connections:
  Rigid Transform3/LConn1 -- B/RConn1
  ReferenceFrame2/RConn1 -- Solid/RConn1 -- Rigid Transform3/RConn1 -- Rigid Transform/LConn1
  F/RConn1 -- Rigid Transform/RConn1

== system non_linear_MBS_PF_final/Test/Arm_long_5_RIGID ==
  ReferenceFrame1 : sm_lib/Frames and Transforms/Reference Frame
  Rigid Transform1 : sm_lib/Frames and Transforms/Rigid Transform  TranslationMethod=Cartesian  TranslationStandardOffset=0  TranslationLengthUnit=m  TranslationStandardAxis=+Z  TranslationCartesianOffset=[0 0 0]  TranslationROffset=0  TranslationROffsetUnits=m  TranslationZOffset=0  TranslationZOffsetUnits=m  TranslationThetaOffset=0  TranslationThetaOffsetUnits=deg  RotationMethod=ArbitraryAxis  RotationAngleUnits=deg  RotationStandardAxis=+Z  RotationAngle=-90  RotationArbitraryAxis=[0 1 0]  FollAlignAxisA=+X  BaseAlignAxisA=+Y  FollAlignAxisB=+Y  BaseAlignAxisB=+Z  RotationSequenceAxes=FollowerAxes  RotationSequence=XYX  RotationSequenceAngles=[0 0 0]  RotationSequenceAnglesUnits=deg  RotationMatrix=[1 0 0; 0 1 0; 0 0 1]
  Rigid Transform2 : sm_lib/Frames and Transforms/Rigid Transform  TranslationMethod=Cartesian  TranslationStandardOffset=0  TranslationLengthUnit=m  TranslationStandardAxis=+Z  TranslationCartesianOffset=[0 0 0]  TranslationROffset=0  TranslationROffsetUnits=m  TranslationZOffset=0  TranslationZOffsetUnits=m  TranslationThetaOffset=0  TranslationThetaOffsetUnits=deg  RotationMethod=ArbitraryAxis  RotationAngleUnits=deg  RotationStandardAxis=+Z  RotationAngle=0  RotationArbitraryAxis=[0 1 0]  FollAlignAxisA=+X  BaseAlignAxisA=+Y  FollAlignAxisB=+Y  BaseAlignAxisB=+Z  RotationSequenceAxes=FollowerAxes  RotationSequence=XYX  RotationSequenceAngles=[0 0 0]  RotationSequenceAnglesUnits=deg  RotationMatrix=[1 0 0; 0 1 0; 0 0 1]
  Solid : sm_lib/Body Elements/File Solid  ExtGeomFileName=Arm_long_Default_sldprt.STEP  UnitType=FromFile  ExtGeomFileUnits=m  StepReaderType=OCC  ExportConvexHullGeometry=off  InertiaType=CalculateFromGeometry  Mass=13.5799  MassUnits=kg  CenterOfMass=smiData.Solid(19).CoM  CenterOfMassUnits=mm  MomentsOfInertia=smiData.Solid(19).MoI  MomentsOfInertiaUnits=kg*mm^2  ProductsOfInertia=smiData.Solid(19).PoI  ProductsOfInertiaUnits=kg*mm^2  BasedOnType=Mass  Density=7800  DensityUnits=kg/m^3  DoExposeReferenceFrame=on  SerializedFrames=<Frames/>  GraphicType=FromGeometry  MarkerShape=Sphere  MarkerSize=10  GraphicVisPropType=SimpleVisualProperties  GraphicDiffuseColor=[0.2 0.6 0.0]  GraphicSpecularColor=[0.5 0.5 0.5 1.0]  GraphicAmbientColor=[0.15 0.15 0.15 1.0]  GraphicEmissiveColor=[0.0 0.0 0.0 1.0]  GraphicShininess=75  GraphicOpacity=smiData.Solid(19).opacity
  F : PMIOPort  Side=Left
  B : PMIOPort  Port=2  Side=Right
  connections:
  Solid/RConn1 -- ReferenceFrame1/RConn1 -- Rigid Transform2/LConn1 -- Rigid Transform1/RConn1
  Rigid Transform1/LConn1 -- F/RConn1
  B/RConn1 -- Rigid Transform2/RConn1

== system non_linear_MBS_PF_final/Test/Arm_short_0_RIGID  ==
  ReferenceFrame1 : sm_lib/Frames and Transforms/Reference Frame
  Rigid Transform : sm_lib/Frames and Transforms/Rigid Transform  TranslationMethod=Cartesian  TranslationStandardOffset=0  TranslationLengthUnit=m  TranslationStandardAxis=+Z  TranslationCartesianOffset=[0 0 0]  TranslationROffset=0  TranslationROffsetUnits=m  TranslationZOffset=0  TranslationZOffsetUnits=m  TranslationThetaOffset=0  TranslationThetaOffsetUnits=deg  RotationMethod=ArbitraryAxis  RotationAngleUnits=deg  RotationStandardAxis=+Z  RotationAngle=90  RotationArbitraryAxis=[0 1 0]  FollAlignAxisA=+X  BaseAlignAxisA=+Y  FollAlignAxisB=+Y  BaseAlignAxisB=+Z  RotationSequenceAxes=FollowerAxes  RotationSequence=XYX  RotationSequenceAngles=[0 0 0]  RotationSequenceAnglesUnits=deg  RotationMatrix=[1 0 0; 0 1 0; 0 0 1]
  Rigid Transform3 : sm_lib/Frames and Transforms/Rigid Transform  TranslationMethod=Cartesian  TranslationStandardOffset=0  TranslationLengthUnit=m  TranslationStandardAxis=+Z  TranslationCartesianOffset=[0 0 0]  TranslationROffset=0  TranslationROffsetUnits=m  TranslationZOffset=0  TranslationZOffsetUnits=m  TranslationThetaOffset=0  TranslationThetaOffsetUnits=deg  RotationMethod=ArbitraryAxis  RotationAngleUnits=deg  RotationStandardAxis=+Z  RotationAngle=0  RotationArbitraryAxis=[1 0 0]  FollAlignAxisA=+X  BaseAlignAxisA=+Y  FollAlignAxisB=+Y  BaseAlignAxisB=+Z  RotationSequenceAxes=FollowerAxes  RotationSequence=XYX  RotationSequenceAngles=[0 0 0]  RotationSequenceAnglesUnits=deg  RotationMatrix=[1 0 0; 0 1 0; 0 0 1]
  Solid : sm_lib/Body Elements/File Solid  ExtGeomFileName=Arm1_Default_sldprt.STEP  UnitType=FromFile  ExtGeomFileUnits=m  StepReaderType=OCC  ExportConvexHullGeometry=off  InertiaType=CalculateFromGeometry  Mass=1.7826  MassUnits=kg  CenterOfMass=smiData.Solid(15).CoM  CenterOfMassUnits=mm  MomentsOfInertia=smiData.Solid(15).MoI  MomentsOfInertiaUnits=kg*mm^2  ProductsOfInertia=smiData.Solid(15).PoI  ProductsOfInertiaUnits=kg*mm^2  BasedOnType=Mass  Density=9000  DensityUnits=kg/m^3  DoExposeReferenceFrame=on  SerializedFrames=<Frames/>  GraphicType=FromGeometry  MarkerShape=Sphere  MarkerSize=10  GraphicVisPropType=SimpleVisualProperties  GraphicDiffuseColor=[0.2 0.6 0.0]  GraphicSpecularColor=[0.5 0.5 0.5 1.0]  GraphicAmbientColor=[0.15 0.15 0.15 1.0]  GraphicEmissiveColor=[0.0 0.0 0.0 1.0]  GraphicShininess=75  GraphicOpacity=smiData.Solid(15).opacity
  B : PMIOPort  Side=Left
  F : PMIOPort  Port=2  Side=Right
  connections:
  Rigid Transform3/LConn1 -- B/RConn1
  ReferenceFrame1/RConn1 -- Rigid Transform/LConn1 -- Solid/RConn1 -- Rigid Transform3/RConn1
  F/RConn1 -- Rigid Transform/RConn1

== system non_linear_MBS_PF_final/Test/Arm_short_1_RIGID1 ==
  ReferenceFrame2 : sm_lib/Frames and Transforms/Reference Frame
  Rigid Transform : sm_lib/Frames and Transforms/Rigid Transform  TranslationMethod=Cartesian  TranslationStandardOffset=0  TranslationLengthUnit=m  TranslationStandardAxis=+Z  TranslationCartesianOffset=[0 0 0.1382]  TranslationROffset=0  TranslationROffsetUnits=m  TranslationZOffset=0  TranslationZOffsetUnits=m  TranslationThetaOffset=0  TranslationThetaOffsetUnits=deg  RotationMethod=ArbitraryAxis  RotationAngleUnits=deg  RotationStandardAxis=+Z  RotationAngle=90  RotationArbitraryAxis=[0 1 0]  FollAlignAxisA=+X  BaseAlignAxisA=+Y  FollAlignAxisB=+Y  BaseAlignAxisB=+Z  RotationSequenceAxes=FollowerAxes  RotationSequence=XYX  RotationSequenceAngles=[0 0 0]  RotationSequenceAnglesUnits=deg  RotationMatrix=[1 0 0; 0 1 0; 0 0 1]
  Rigid Transform3 : sm_lib/Frames and Transforms/Rigid Transform  TranslationMethod=Cartesian  TranslationStandardOffset=0  TranslationLengthUnit=m  TranslationStandardAxis=+Z  TranslationCartesianOffset=[0 0 0]  TranslationROffset=0  TranslationROffsetUnits=m  TranslationZOffset=0  TranslationZOffsetUnits=m  TranslationThetaOffset=0  TranslationThetaOffsetUnits=deg  RotationMethod=ArbitraryAxis  RotationAngleUnits=deg  RotationStandardAxis=+Z  RotationAngle=-90  RotationArbitraryAxis=[0 1 0]  FollAlignAxisA=+X  BaseAlignAxisA=+Y  FollAlignAxisB=+Y  BaseAlignAxisB=+Z  RotationSequenceAxes=FollowerAxes  RotationSequence=XYX  RotationSequenceAngles=[0 0 0]  RotationSequenceAnglesUnits=deg  RotationMatrix=[1 0 0; 0 1 0; 0 0 1]
  Solid1 : sm_lib/Body Elements/File Solid  ExtGeomFileName=Arm2_Default_sldprt.STEP  UnitType=FromFile  ExtGeomFileUnits=m  StepReaderType=HEX  ExportConvexHullGeometry=off  InertiaType=Custom  Mass=smiData.Solid(20).mass  MassUnits=kg  CenterOfMass=smiData.Solid(20).CoM  CenterOfMassUnits=mm  MomentsOfInertia=smiData.Solid(20).MoI  MomentsOfInertiaUnits=kg*mm^2  ProductsOfInertia=smiData.Solid(20).PoI  ProductsOfInertiaUnits=kg*mm^2  BasedOnType=Density  Density=1000  DensityUnits=kg/m^3  DoExposeReferenceFrame=on  SerializedFrames=<Frames/>  GraphicType=FromGeometry  MarkerShape=Sphere  MarkerSize=10  GraphicVisPropType=SimpleVisualProperties  GraphicDiffuseColor=[0.2 0.6 0.0]  GraphicSpecularColor=[0.5 0.5 0.5 1.0]  GraphicAmbientColor=[0.15 0.15 0.15 1.0]  GraphicEmissiveColor=[0.0 0.0 0.0 1.0]  GraphicShininess=75  GraphicOpacity=smiData.Solid(20).opacity
  B : PMIOPort  Side=Left
  F : PMIOPort  Port=2  Side=Right
  connections:
  Rigid Transform/LConn1 -- ReferenceFrame2/RConn1 -- Rigid Transform3/RConn1 -- Solid1/RConn1
  Rigid Transform/RConn1 -- F/RConn1
  B/RConn1 -- Rigid Transform3/LConn1

== system non_linear_MBS_PF_final/Test/Arm_short_2_RIGID ==
  ReferenceFrame2 : sm_lib/Frames and Transforms/Reference Frame
  Rigid Transform : sm_lib/Frames and Transforms/Rigid Transform  TranslationMethod=Cartesian  TranslationStandardOffset=0  TranslationLengthUnit=m  TranslationStandardAxis=+Z  TranslationCartesianOffset=[0 0 0.1382]  TranslationROffset=0  TranslationROffsetUnits=m  TranslationZOffset=0  TranslationZOffsetUnits=m  TranslationThetaOffset=0  TranslationThetaOffsetUnits=deg  RotationMethod=ArbitraryAxis  RotationAngleUnits=deg  RotationStandardAxis=+Z  RotationAngle=90  RotationArbitraryAxis=[0 1 0]  FollAlignAxisA=+X  BaseAlignAxisA=+Y  FollAlignAxisB=+Y  BaseAlignAxisB=+Z  RotationSequenceAxes=FollowerAxes  RotationSequence=XYX  RotationSequenceAngles=[0 0 0]  RotationSequenceAnglesUnits=deg  RotationMatrix=[1 0 0; 0 1 0; 0 0 1]
  Rigid Transform3 : sm_lib/Frames and Transforms/Rigid Transform  TranslationMethod=Cartesian  TranslationStandardOffset=0  TranslationLengthUnit=m  TranslationStandardAxis=+Z  TranslationCartesianOffset=[0 0 0]  TranslationROffset=0  TranslationROffsetUnits=m  TranslationZOffset=0  TranslationZOffsetUnits=m  TranslationThetaOffset=0  TranslationThetaOffsetUnits=deg  RotationMethod=ArbitraryAxis  RotationAngleUnits=deg  RotationStandardAxis=+Z  RotationAngle=-90  RotationArbitraryAxis=[0 1 0]  FollAlignAxisA=+X  BaseAlignAxisA=+Y  FollAlignAxisB=+Y  BaseAlignAxisB=+Z  RotationSequenceAxes=FollowerAxes  RotationSequence=XYX  RotationSequenceAngles=[0 0 0]  RotationSequenceAnglesUnits=deg  RotationMatrix=[1 0 0; 0 1 0; 0 0 1]
  Solid : sm_lib/Body Elements/File Solid  ExtGeomFileName=Arm_short_Default_sldprt.STEP  UnitType=FromFile  ExtGeomFileUnits=m  StepReaderType=OCC  ExportConvexHullGeometry=off  InertiaType=CalculateFromGeometry  Mass=3.0795  MassUnits=kg  CenterOfMass=smiData.Solid(18).CoM  CenterOfMassUnits=mm  MomentsOfInertia=smiData.Solid(18).MoI  MomentsOfInertiaUnits=kg*mm^2  ProductsOfInertia=smiData.Solid(18).PoI  ProductsOfInertiaUnits=kg*mm^2  BasedOnType=Mass  Density=1000  DensityUnits=kg/m^3  DoExposeReferenceFrame=on  SerializedFrames=<Frames/>  GraphicType=FromGeometry  MarkerShape=Sphere  MarkerSize=10  GraphicVisPropType=SimpleVisualProperties  GraphicDiffuseColor=[0.2 0.6 0.0]  GraphicSpecularColor=[0.5 0.5 0.5 1.0]  GraphicAmbientColor=[0.15 0.15 0.15 1.0]  GraphicEmissiveColor=[0.0 0.0 0.0 1.0]  GraphicShininess=75  GraphicOpacity=smiData.Solid(18).opacity
  B : PMIOPort  Side=Left
  F : PMIOPort  Port=2  Side=Right
  connections:
  Rigid Transform/LConn1 -- Solid/RConn1 -- ReferenceFrame2/RConn1 -- Rigid Transform3/RConn1
  Rigid Transform/RConn1 -- F/RConn1
  Rigid Transform3/LConn1 -- B/RConn1

== system non_linear_MBS_PF_final/Test/Arm_short_3_RIGID ==
  ReferenceFrame2 : sm_lib/Frames and Transforms/Reference Frame
  Rigid Transform : sm_lib/Frames and Transforms/Rigid Transform  TranslationMethod=Cartesian  TranslationStandardOffset=0  TranslationLengthUnit=m  TranslationStandardAxis=+Z  TranslationCartesianOffset=[0 0 0.1382]  TranslationROffset=0  TranslationROffsetUnits=m  TranslationZOffset=0  TranslationZOffsetUnits=m  TranslationThetaOffset=0  TranslationThetaOffsetUnits=deg  RotationMethod=ArbitraryAxis  RotationAngleUnits=deg  RotationStandardAxis=+Z  RotationAngle=90  RotationArbitraryAxis=[0 1 0]  FollAlignAxisA=+X  BaseAlignAxisA=+Y  FollAlignAxisB=+Y  BaseAlignAxisB=+Z  RotationSequenceAxes=FollowerAxes  RotationSequence=XYX  RotationSequenceAngles=[0 0 0]  RotationSequenceAnglesUnits=deg  RotationMatrix=[1 0 0; 0 1 0; 0 0 1]
  Rigid Transform3 : sm_lib/Frames and Transforms/Rigid Transform  TranslationMethod=Cartesian  TranslationStandardOffset=0  TranslationLengthUnit=m  TranslationStandardAxis=+Z  TranslationCartesianOffset=[0 0 0]  TranslationROffset=0  TranslationROffsetUnits=m  TranslationZOffset=0  TranslationZOffsetUnits=m  TranslationThetaOffset=0  TranslationThetaOffsetUnits=deg  RotationMethod=ArbitraryAxis  RotationAngleUnits=deg  RotationStandardAxis=+Z  RotationAngle=-90  RotationArbitraryAxis=[0 1 0]  FollAlignAxisA=+X  BaseAlignAxisA=+Y  FollAlignAxisB=+Y  BaseAlignAxisB=+Z  RotationSequenceAxes=FollowerAxes  RotationSequence=XYX  RotationSequenceAngles=[0 0 0]  RotationSequenceAnglesUnits=deg  RotationMatrix=[1 0 0; 0 1 0; 0 0 1]
  Solid : sm_lib/Body Elements/File Solid  ExtGeomFileName=Arm_short_Default_sldprt.STEP  UnitType=FromFile  ExtGeomFileUnits=m  StepReaderType=OCC  ExportConvexHullGeometry=off  InertiaType=CalculateFromGeometry  Mass=3.0795  MassUnits=kg  CenterOfMass=smiData.Solid(18).CoM  CenterOfMassUnits=mm  MomentsOfInertia=smiData.Solid(18).MoI  MomentsOfInertiaUnits=kg*mm^2  ProductsOfInertia=smiData.Solid(18).PoI  ProductsOfInertiaUnits=kg*mm^2  BasedOnType=Mass  Density=1000  DensityUnits=kg/m^3  DoExposeReferenceFrame=on  SerializedFrames=<Frames/>  GraphicType=FromGeometry  MarkerShape=Sphere  MarkerSize=10  GraphicVisPropType=SimpleVisualProperties  GraphicDiffuseColor=[0.2 0.6 0.0]  GraphicSpecularColor=[0.5 0.5 0.5 1.0]  GraphicAmbientColor=[0.15 0.15 0.15 1.0]  GraphicEmissiveColor=[0.0 0.0 0.0 1.0]  GraphicShininess=75  GraphicOpacity=smiData.Solid(18).opacity
  B : PMIOPort  Side=Left
  F : PMIOPort  Port=2  Side=Right
  connections:
  Rigid Transform3/LConn1 -- B/RConn1
  Rigid Transform/LConn1 -- Solid/RConn1 -- ReferenceFrame2/RConn1 -- Rigid Transform3/RConn1
  Rigid Transform/RConn1 -- F/RConn1
